simulink model "TosAeb"  (R2021a)
settings: Solver=FixedStepDiscrete  StartTime=0.0  StopTime=inf  FixedStep=0.01  RelTol=1e-3
undefined symbols (not defined in the file or in its folder): ACCModSts, AccSts1, AEB_ObjectMovingOrientation, AEB_ObjectMovingState, CN_BTCActive, CN_CurrentGearR, CN_CurrentGearR_DHT, CN_PTCActive, CN_VDCActive, CN_VSC_EspOff, CN_VSC_FCW_AEB_Error, CN_VSC_FCW_AEB_NoDispay, CN_VSC_FCW_AEB_PerfmDgdtn, CN_VSC_FCW_AEB_TempUnAvlbl, CN_VSC_VehStandstill, CNaccelAvoidMax, CNaccelAvoidMin, CNbrakePedalPress, CNcomfortStrLeftAccelMax, CNcomfortStrLeftAccelMin, CNcomfortStrRightAccelMax, CNcomfortStrRightAccelMin, CNDrvDoorOpen, CNDrvModeEco, CNDrvModeNorm, CNDrvModeOther, CNDrvModeSport, CNDrvSBRreminder, CNNull, CNobjInvalidID, CNPassDoorOpen, CNPassSBRreminder, CNPEBSAccelNegInf, CNPEBSAccelNull, CNPEBSTimeInf, CNPEBSTimeNull, CNSTOPMAX, CNSTOPNULL, CNsubjWidth, CNSwtOFF, CNSwtON, CNTTCMAX, CNTTCNULL, CNVehConfigAEBCycNone, CNVehConfigAEBNone, CNVehConfigAEBPedNone, CNVehConfigFCWNone, CNVehConfigJANone, CollisionSeverity, FALSE, FCWSnvty, JACycObjCalcInfoDefaultParams, JAPedObjCalcInfoDefaultParams, JAVehObjCalcInfoDefaultParams, latCycObjCalcInfoDefaultParams, latPedObjCalcInfoDefaultParams, longObjCalcInfoDefaultParams, ModSts, movingStatus, ObjectType ... (+1112)
libraries: simulink x3031, AEBSFunctionLib x50, Ta_Library x33, AEBSStateMachineLib x23, Mylibrary x12, simulink_extras x7, ObjSelect_lib x3, dspstat3 x2
(part 5 of 102)

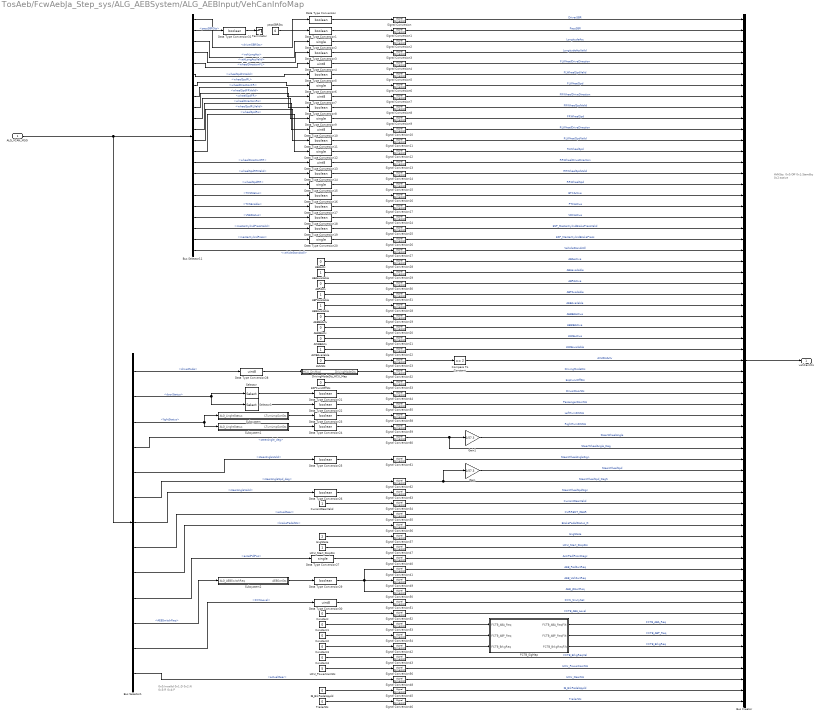
== system TosAeb/FcwAebJa_Step_sys/ALG_AEBSystem/ALG_AEBInput/VehCanInfoMap ==
  note: AVHSts:
      | 0x0:Off
      | 0x1:Standby
      | 0x2:active
  note: 0x0:Invailid
      | 0x1:D
      | 0x2:N
      | 0x3:R
      | 0x4:P
  ALG_VCAN_MSG : Inport  OutDataTypeStr=Bus: VehicleInfo_Struct  PortDimensions=1  BusOutputAsStruct=on
  ABAactv : Constant  Value=0  OutDataTypeStr=boolean
  ABAavailable : Constant  OutDataTypeStr=boolean
  ABPActv : Constant  Value=0  OutDataTypeStr=boolean
  ABPAvailable : Constant  OutDataTypeStr=boolean
  AEBAvailable : Constant  OutDataTypeStr=boolean
  AEBBAActv : Constant  Value=0  OutDataTypeStr=boolean
  AEBIBActv : Constant  Value=0  OutDataTypeStr=boolean
  AVHSts : Constant  Value=0  OutDataTypeStr=boolean
  AWBAvailable : Constant  OutDataTypeStr=boolean
  AWBBActv : Constant  Value=0  OutDataTypeStr=boolean
  Bus Creator : BusCreator  Inputs=63  OutDataTypeStr=Bus: BusVehCanInfo
      settings: InheritFromInputs=off
  Bus Selector1 : BusSelector  OutputSignals=bodyInfo.driveMode,bodyInfo.doorStatus,bodyInfo.lightStatus,chasssisInfo.steerAngle_deg,chasssisInfo.steerAngleValid,chasssisInfo.steerAngleSpd_deg,chasssisInfo.steerAngleValid,chasssisInfo.actualGear,chasssisInfo.brakePedalSts,chasssisInfo.accelPdlPos,bodyInfo.AEBSwitchReq,bodyInfo.FCWLevel,chasssisInfo.actualGear
  Bus Selector11 : BusSelector  OutputSignals=bodyInfo.driverSBRSts,bodyInfo.passSBRSts,chasssisInfo.vehLongAcc,chasssisInfo.vehLongAccValid,chasssisInfo.wheelDirectionFL,chasssisInfo.wheelSpdFLValid,chasssisInfo.wheelSpdFL,chasssisInfo.wheelDirectionFR,chasssisInfo.wheelSpdFRValid,chasssisInfo.wheelSpdFR,chasssisInfo.wheelDirectionRL,chasssisInfo.wheelSpdRLValid,chasssisInfo.wheelSpdRL,chasssisInfo.wheelDirectionRR,chasssisInfo.wheelSpdRRValid,chasssisInfo.wheelSpdRR,chasssisInfo.TCSStatus,chasssisInfo.TCSEnable,chasssisInfo.VSEStatus,chasssisInfo.masterCylindPressValid,chasssisInfo.masterCylindPress,chasssisInfo.vehicleStandstill
  Compare To Constant : simulink/Logic and Bit Operations/Compare To Constant  relop===  const=2  OutDataTypeStr=boolean
  Constant{,1..3} (4 alike) : Constant  Value=0  OutDataTypeStr=uint8
  Constant4 : Constant  Value=0  OutDataTypeStr=single
  CurrentGearValid : Constant  OutDataTypeStr=boolean
  Data Type Conversion{,1,3,5,8,11,14,16..19,21..26,29,31} (19 alike) : DataTypeConversion  OutDataTypeStr=boolean
  Data Type Conversion{4,7,10,13,28,30} (6 alike) : DataTypeConversion  OutDataTypeStr=uint8
  Data Type Conversion{2,6,9,12,15,20,27} (7 alike) : DataTypeConversion  OutDataTypeStr=single
  DrivingModeDis_HCU_Map : Subsystem  VariantControl=P_AEB_PowerTrainConfig == 1
  ESPFuncOffSts : Constant  Value=0  OutDataTypeStr=boolean
  EngState : Constant  Value=2  OutDataTypeStr=uint8
  FCTB_SigMap : Subsystem
  Gain : Gain  Gain=1/57.3
  Gain1 : Gain  Gain=1/57.3
  HCU_PowertrainSts : Constant  Value=5  OutDataTypeStr=uint8
  HCU_Start_StopSts : Constant  OutDataTypeStr=uint8
  IB_BrkPedalAppld : Constant  Value=0  OutDataTypeStr=boolean
  Selector : Selector  InputPortWidth=6  IndexOptions=Index vector (dialog)  Indices=1  OutputSizes=1
  Selector1 : Selector  InputPortWidth=6  IndexOptions=Index vector (dialog)  Indices=2  OutputSizes=1
  Signal Conversion{,1..37,40..56,58..63} (61 alike) : SignalConversion  OverrideOpt=off
  Subsystem : Subsystem
  Subsystem1 : Subsystem
  Subsystem2 : Subsystem
  Terminator : Terminator
  TrailerSts : Constant  Value=0  OutDataTypeStr=boolean
  passSBRSts : Constant  Value=0  OutDataTypeStr=boolean
  vehCanInfo : Outport  OutDataTypeStr=Bus: BusVehCanInfo
  wires:
  Bus Selector1/2 -> Selector1/1, Selector/1  "<doorStatus>"
  Subsystem2/1(AEBSwtSts) -> Data Type Conversion29/1
  Signal Conversion56/1 -> Bus Creator/60  "HCU_PowertrainSts"
  Data Type Conversion30/1 -> Signal Conversion51/1
  Bus Selector11/5 -> Data Type Conversion4/1  "<wheelDirectionFL>"
  Bus Selector1/12 -> Data Type Conversion30/1  "<FCWLevel>"
  Bus Selector1/11 -> Subsystem2/1(ALG_AEBSwitchReq)  "<AEBSwitchReq>"
  CurrentGearValid/1 -> Signal Conversion34/1
  Bus Selector1/9 -> Signal Conversion36/1  "<brakePedalSts>"
  Data Type Conversion21/1 -> Signal Conversion44/1
  Bus Selector1/1 -> Data Type Conversion28/1  "<driveMode>"
  Bus Selector11/10 -> Data Type Conversion9/1  "<wheelSpdFR>"
  Bus Selector11/19 -> Data Type Conversion18/1  "<VSEStatus>"
  Data Type Conversion27/1 -> Signal Conversion40/1
  Bus Selector1/13 -> Signal Conversion48/1  "<actualGear>"
  Bus Selector1/10 -> Data Type Conversion27/1  "<accelPdlPos>"
  Data Type Conversion24/1 -> Signal Conversion59/1
  Bus Selector11/13 -> Data Type Conversion12/1  "<wheelSpdRL>"
  Data Type Conversion26/1 -> Signal Conversion63/1
  Data Type Conversion15/1 -> Signal Conversion15/1
  Signal Conversion25/1 -> Bus Creator/20  "ESP_MasterCylindBrakePressValid"
  Data Type Conversion16/1 -> Signal Conversion16/1
  Data Type Conversion25/1 -> Signal Conversion61/1
  Data Type Conversion2/1 -> Signal Conversion2/1
  IB_BrkPedalAppld/1 -> Signal Conversion45/1
  Data Type Conversion12/1 -> Signal Conversion12/1
  Signal Conversion34/1 -> Bus Creator/45  "CurrentGearValid"
  Data Type Conversion23/1 -> Signal Conversion58/1
  Data Type Conversion22/1 -> Signal Conversion55/1
  HCU_PowertrainSts/1 -> Signal Conversion56/1
  Data Type Conversion20/1 -> Signal Conversion26/1
  HCU_Start_StopSts/1 -> Signal Conversion47/1
  EngState/1 -> Signal Conversion37/1
  Bus Selector1/6 -> Signal Conversion62/1  "<steerAngleSpd_deg>"
  Bus Selector1/4 -> Signal Conversion60/1  "<steerAngle_deg>"
  Bus Selector1/5 -> Data Type Conversion25/1  "<steerAngleValid>"
  Subsystem1/1(LTurnLmpSwtSts) -> Data Type Conversion24/1
  Subsystem/1(LTurnLmpSwtSts) -> Data Type Conversion23/1
  Data Type Conversion5/1 -> Signal Conversion5/1
  Signal Conversion16/1 -> Bus Creator/17  "BTCActive"
  Data Type Conversion18/1 -> Signal Conversion24/1
  Data Type Conversion19/1 -> Signal Conversion25/1
  Signal Conversion15/1 -> Bus Creator/16  "RRWheelSpd"
  Bus Selector11/7 -> Data Type Conversion6/1  "<wheelSpdFL>"
  Signal Conversion32/1 -> Bus Creator/33  "DrivingModeDis"
  Bus Selector11/15 -> Data Type Conversion14/1  "<wheelSpdRRValid>"
  Data Type Conversion4/1 -> Signal Conversion4/1
  Data Type Conversion17/1 -> Signal Conversion17/1
  Data Type Conversion7/1 -> Signal Conversion7/1
  Signal Conversion5/1 -> Bus Creator/6  "FLWheelSpdValid"
  Data Type Conversion14/1 -> Signal Conversion14/1
  Signal Conversion23/1 -> Compare To Constant/1
  Data Type Conversion28/1 -> DrivingModeDis_HCU_Map/1(HCU_DrvMod)
  Data Type Conversion10/1 -> Signal Conversion10/1
  Data Type Conversion13/1 -> Signal Conversion13/1
  Data Type Conversion11/1 -> Signal Conversion11/1
  Data Type Conversion8/1 -> Signal Conversion8/1
  Data Type Conversion9/1 -> Signal Conversion9/1
  Bus Selector11/21 -> Data Type Conversion20/1  "<masterCylindPress>"
  Data Type Conversion3/1 -> Signal Conversion3/1
  Data Type Conversion6/1 -> Signal Conversion6/1
  Data Type Conversion1/1 -> Signal Conversion1/1
  TrailerSts/1 -> Signal Conversion46/1
  Data Type Conversion/1 -> Signal Conversion/1
  Bus Selector11/11 -> Data Type Conversion10/1  "<wheelDirectionRL>"
  Bus Selector11/18 -> Data Type Conversion17/1  "<TCSEnable>"
  Selector1/1 -> Data Type Conversion22/1
  Bus Selector11/20 -> Data Type Conversion19/1  "<masterCylindPressValid>"
  Bus Selector11/22 -> Signal Conversion27/1  "<vehicleStandstill>"
  Bus Selector11/14 -> Data Type Conversion13/1  "<wheelDirectionRR>"
  Bus Selector11/16 -> Data Type Conversion15/1  "<wheelSpdRR>"
  Bus Selector11/17 -> Data Type Conversion16/1  "<TCSStatus>"
  Bus Selector11/12 -> Data Type Conversion11/1  "<wheelSpdRLValid>"
  Bus Selector11/8 -> Data Type Conversion7/1  "<wheelDirectionFR>"
  Signal Conversion48/1 -> Bus Creator/61  "HCU_GearSts"
  Bus Selector11/9 -> Data Type Conversion8/1  "<wheelSpdFRValid>"
  Bus Selector11/6 -> Data Type Conversion5/1  "<wheelSpdFLValid>"
  Bus Selector11/4 -> Data Type Conversion3/1  "<vehLongAccValid>"
  Bus Selector11/3 -> Data Type Conversion2/1  "<vehLongAcc>"
  Bus Selector11/1 -> Data Type Conversion/1  "<driverSBRSts>"
  ESPFuncOffSts/1 -> Signal Conversion33/1
  AVHSts/1 -> Signal Conversion23/1
  AWBBActv/1 -> Signal Conversion21/1
  AEBIBActv/1 -> Signal Conversion20/1
  AEBBAActv/1 -> Signal Conversion19/1
  AWBAvailable/1 -> Signal Conversion22/1
  AEBAvailable/1 -> Signal Conversion18/1
  ABPAvailable/1 -> Signal Conversion31/1
  ABPActv/1 -> Signal Conversion30/1
  ABAavailable/1 -> Signal Conversion29/1
  ABAactv/1 -> Signal Conversion28/1
  Constant4/1 -> Signal Conversion43/1
  Signal Conversion13/1 -> Bus Creator/14  "RRWheelDriveDirection"
  Constant3/1 -> Signal Conversion42/1
  Signal Conversion47/1 -> Bus Creator/49  "HCU_Start_StopSts"
  Signal Conversion46/1 -> Bus Creator/63  "TrailerSts"
  Signal Conversion43/1 -> Bus Creator/59  "FCTB_BrkgReqVal"
  Signal Conversion42/1 -> FCTB_SigMap/3(FCTB_BrkgReq)
  FCTB_SigMap/3(FCTB_BrkgReqFilt) -> Bus Creator/58  "FCTB_BrkgReq"
  FCTB_SigMap/1(FCTB_ABA_ReqFilt) -> Bus Creator/56  "FCTB_ABA_Req"
  Data Type Conversion29/1 -> Signal Conversion41/1, Signal Conversion50/1, Signal Conversion49/1
  FCTB_SigMap/2(FCTB_ABP_ReqFilt) -> Bus Creator/57  "FCTB_ABP_Req"
  Bus Selector1/3 -> Subsystem1/1(ALG_LinghtStatus), Subsystem/1(ALG_LinghtStatus)  "<lightStatus>"
  DrivingModeDis_HCU_Map/1(DrivingModeDis) -> Signal Conversion32/1
  Gain1/1 -> Bus Creator/39  "SteerWheelAngle"
  Gain/1 -> Bus Creator/42  "SteerWheelSpd"
  Compare To Constant/1 -> Bus Creator/32  "AVHStsActv"
  Signal Conversion61/1 -> Bus Creator/41  "SteerWheelAngleSign"
  Signal Conversion63/1 -> Bus Creator/44  "SteerWheelSpdSign"
  Signal Conversion58/1 -> Bus Creator/37  "LeftTurnSWSts"
  Signal Conversion59/1 -> Bus Creator/38  "RightTurnSWSts"
  Signal Conversion54/1 -> FCTB_SigMap/2(FCTB_ABP_Req)
  Signal Conversion55/1 -> Bus Creator/36  "PassengerDoorSts"
  Signal Conversion52/1 -> Bus Creator/55  "FCTB_ABA_Level"
  Signal Conversion53/1 -> FCTB_SigMap/1(FCTB_ABA_Req)
  Signal Conversion49/1 -> Bus Creator/52  "AEB_VehSwtReq"
  Signal Conversion30/1 -> Bus Creator/25  "ABPActive"
  Signal Conversion50/1 -> Bus Creator/53  "AEB_JASwtReq"
  Signal Conversion51/1 -> Bus Creator/54  "FCW_SnvtySet"
  Signal Conversion44/1 -> Bus Creator/35  "DriverDoorSts"
  Signal Conversion45/1 -> Bus Creator/62  "IB_BrkPedalAppld"
  Signal Conversion40/1 -> Bus Creator/50  "AccrPedlPosnDiagc"
  Signal Conversion60/1 -> Bus Creator/40, Gain1/1  "SteerWheelAngle_Deg"
  Signal Conversion41/1 -> Bus Creator/51  "AEB_PedSwtReq"
  Signal Conversion36/1 -> Bus Creator/47  "BrakePedalStatus_G"
  Signal Conversion37/1 -> Bus Creator/48  "EngState"
  Signal Conversion33/1 -> Bus Creator/34  "EspFuncOffSts"
  Signal Conversion35/1 -> Bus Creator/46  "CURRENT_GEAR"
  Signal Conversion31/1 -> Bus Creator/26  "ABPAvailable"
  Signal Conversion29/1 -> Bus Creator/24  "ABAavailable"
  Signal Conversion27/1 -> Bus Creator/22  "VehicleStandstill"
  Signal Conversion28/1 -> Bus Creator/23  "ABAactive"
  Signal Conversion26/1 -> Bus Creator/21  "ESP_MasterCylindBrakePress"
  Signal Conversion22/1 -> Bus Creator/31  "AWBavailable"
  Signal Conversion24/1 -> Bus Creator/19  "VDCActive"
  Signal Conversion21/1 -> Bus Creator/30  "AWBactive"
  Signal Conversion20/1 -> Bus Creator/29  "AEBIBActive"
  Signal Conversion18/1 -> Bus Creator/27  "AEBAvailable"
  Signal Conversion19/1 -> Bus Creator/28  "AEBBAActive"
  Signal Conversion17/1 -> Bus Creator/18  "PTCActive"
  Signal Conversion9/1 -> Bus Creator/10  "FRWheelSpd"
  Signal Conversion8/1 -> Bus Creator/9  "FRWheelSpdValid"
  Signal Conversion14/1 -> Bus Creator/15  "RRWheelSpdValid"
  Signal Conversion12/1 -> Bus Creator/13  "RLWheelSpd"
  Signal Conversion10/1 -> Bus Creator/11  "RLWheelDriveDirection"
  Signal Conversion11/1 -> Bus Creator/12  "RLWheelSpdValid"
  Signal Conversion6/1 -> Bus Creator/7  "FLWheelSpd"
  Signal Conversion4/1 -> Bus Creator/5  "FLWheelDriveDirection"
  Signal Conversion7/1 -> Bus Creator/8  "FRWheelDriveDirection"
  Signal Conversion2/1 -> Bus Creator/3  "LongitudeAcc"
  Signal Conversion3/1 -> Bus Creator/4  "LongitudeAccValid"
  Signal Conversion1/1 -> Bus Creator/2  "PassSBR"
  Signal Conversion/1 -> Bus Creator/1  "DriverSBR"
  Bus Creator/1 -> vehCanInfo/1
  Constant2/1 -> Signal Conversion54/1
  Constant1/1 -> Signal Conversion53/1
  Constant/1 -> Signal Conversion52/1
  Bus Selector1/8 -> Signal Conversion35/1  "<actualGear>"
  Bus Selector1/7 -> Data Type Conversion26/1  "<steerAngleValid>"
  Selector/1 -> Data Type Conversion21/1
  ALG_VCAN_MSG/1 -> Bus Selector1/1, Bus Selector11/1
  Signal Conversion62/1 -> Bus Creator/43, Gain/1  "SteerWheelSpd_DegS"
  Bus Selector11/2 -> Data Type Conversion31/1  "<passSBRSts>"
  passSBRSts/1 -> Data Type Conversion1/1
  Data Type Conversion31/1 -> Terminator/1

== system TosAeb/FcwAebJa_Step_sys/ALG_AEBSystem/ALG_AEBInput/VehConfigInfoMap ==
  Bus Creator : BusCreator  Inputs=6  OutDataTypeStr=Bus: BusVehConfigInfo
      settings: InheritFromInputs=off
  Constant{,1..5} (6 alike) : Constant  OutDataTypeStr=boolean
  Data Type Conversion{,1..5} (6 alike) : DataTypeConversion  OutDataTypeStr=boolean
  vehConfigInfo : Outport
  wires:
  Data Type Conversion5/1 -> Bus Creator/6
  Constant5/1 -> Data Type Conversion5/1
  Constant/1 -> Data Type Conversion1/1
  Constant1/1 -> Data Type Conversion/1
  Constant2/1 -> Data Type Conversion3/1
  Constant3/1 -> Data Type Conversion2/1
  Constant4/1 -> Data Type Conversion4/1
  Bus Creator/1 -> vehConfigInfo/1
  Data Type Conversion4/1 -> Bus Creator/5
  Data Type Conversion2/1 -> Bus Creator/4
  Data Type Conversion3/1 -> Bus Creator/3
  Data Type Conversion/1 -> Bus Creator/2
  Data Type Conversion1/1 -> Bus Creator/1

== system TosAeb/FcwAebJa_Step_sys/ALG_AEBSystem/ALG_AEBInput/subjInfoMap ==
  vxvRefMsFilt : Inport
  axvRefMs2 : Inport  Port=2
  ayvRefMs2 : Inport  Port=3
  kapTraj : Inport  Port=4
  psiDtOpt : Inport  Port=5
  Bus Creator : BusCreator  Inputs=5  OutDataTypeStr=Bus: BusSubjInfo
      settings: InheritFromInputs=off
  Signal Conversion{,1..4} (5 alike) : SignalConversion  OverrideOpt=off
  subjInfo : Outport  OutDataTypeStr=Bus: BusSubjInfo
  wires:
  Signal Conversion/1 -> Bus Creator/1  "subjVel"
  Signal Conversion1/1 -> Bus Creator/2  "subjLongAccel"
  Signal Conversion2/1 -> Bus Creator/3  "subjLatAccel"
  Signal Conversion3/1 -> Bus Creator/4  "subjCurvTraj"
  Signal Conversion4/1 -> Bus Creator/5  "subjYawRate"
  Bus Creator/1 -> subjInfo/1
  vxvRefMsFilt/1 -> Signal Conversion/1  "subjVel"
  axvRefMs2/1 -> Signal Conversion1/1  "subjLongAccel"
  psiDtOpt/1 -> Signal Conversion4/1  "subjYawRate"
  ayvRefMs2/1 -> Signal Conversion2/1  "subjLatAccel"
  kapTraj/1 -> Signal Conversion3/1  "subjCurvTraj"

== system TosAeb/FcwAebJa_Step_sys/ALG_AEBSystem/ALG_AEBInput/vxvRefMsFilt ==
  vxvRefMs : Inport  OutDataTypeStr=single
  VehSpd : Inport  Port=2
  Add : Sum  Inputs=+++
  Constant : Constant  Value=P_AEB_vxvref_Config
  Gain1 : Gain  Gain=1/3
  Switch : Switch  Criteria=u2 ~= 0
  Unit Delay : UnitDelay  SampleTime=-1  HasFrameUpgradeWarning=on
  Unit Delay1 : UnitDelay  SampleTime=-1  HasFrameUpgradeWarning=on
  vxvRefMsFilt : Outport
  wires:
  VehSpd/1 -> Unit Delay/1, Add/1
  Unit Delay/1 -> Unit Delay1/1, Add/2
  Unit Delay1/1 -> Add/3
  Add/1 -> Gain1/1
  Constant/1 -> Switch/2
  Gain1/1 -> Switch/3  "AEB_vxvRefMs_Can"
  vxvRefMs/1 -> Switch/1
  Switch/1 -> vxvRefMsFilt/1  "AEB_vxvRefMsFilt"

== system TosAeb/FcwAebJa_Step_sys/ALG_AEBSystem/ALG_AEBOutput/AEBS_BrkInfoCalc ==
  brkInfoInputSignals : Inport
  AEBS_BrkInfoMap : Subsystem
  AEB_BrkReqFuncCalc : Subsystem
  AEB_VehHldReqCalc : Subsystem
  Bus Selector : BusSelector  OutputSignals=AEBpartFuncImpArb,AEBfullFuncImpArb,EBAFuncImpArb,cycAEBpartFuncImpArb,cycAEBfullFuncImpArb,pedAEBfullFuncImpArb,JA_AEBfullFuncImpArb,JA_pedAEBRawModSts,FCTBFuncImpArb
  Bus Selector1 : BusSelector  OutputSignals=vehAEBpartRawModSts,vehAEBfullRawModSts,vehEBARawModSts,pedAEBfullRawModSts,cycAEBpartRawModSts,cycAEBfullRawModSts,JA_vehAEBRawModSts,JA_pedAEBRawModSts,AEBTrigger,JA_cycAEBRawModSts
  Bus Selector2 : BusSelector  OutputSignals=AEBTrigger,EBAFuncImpArb
  Bus Selector3 : BusSelector  OutputSignals=ABALvl,ABATrigger,AEBTrigger,axTgtReqValue,acuteWarningTrigger,acuteWarningLvl,ABPTrigger
  EBA_TgtDecel_ReqJudge : Subsystem
  AEB_BRK_INFO : Outport  OutDataTypeStr=Bus: AEB_BRK_INFO_BUS
  wires:
  AEBS_BrkInfoMap/1(AEB_BRK_INFO) -> AEB_BRK_INFO/1
  Bus Selector3/7 -> AEBS_BrkInfoMap/7(ABPTrigger)  "<ABPTrigger>"
  Bus Selector3/6 -> AEBS_BrkInfoMap/6(acuteWarningLvl)  "<acuteWarningLvl>"
  Bus Selector3/5 -> AEBS_BrkInfoMap/5(acuteWarningTrigger)  "<acuteWarningTrigger>"
  Bus Selector3/4 -> AEBS_BrkInfoMap/4(axTgtReqValue)  "<axTgtReqValue>"
  Bus Selector3/3 -> AEBS_BrkInfoMap/3(AEBTrigger)  "<AEBTrigger>"
  Bus Selector3/1 -> AEBS_BrkInfoMap/1(ABALvl)  "<ABALvl>"
  Bus Selector3/2 -> AEBS_BrkInfoMap/2(ABATrigger)  "<ABATrigger>"
  Bus Selector2/1 -> EBA_TgtDecel_ReqJudge/1(AEBtrigger)  "<AEBTrigger>"
  Bus Selector2/2 -> EBA_TgtDecel_ReqJudge/2(EBAFuncImpArb)  "<EBAFuncImpArb>"
  EBA_TgtDecel_ReqJudge/1(Inter_EBA_TgtDecel_Req) -> AEBS_BrkInfoMap/8(EBA_TgtDecel_Req)  "EBA_TgtDecel_Req"
  AEB_VehHldReqCalc/1(Inter_AEB_VehHldReq) -> AEBS_BrkInfoMap/9(AEB_VehHldReq)  "AEB_VehHldReq"
  AEB_BrkReqFuncCalc/1(AEB_BrkReqFunc) -> AEBS_BrkInfoMap/10(AEB_BrkReqFunc)  "AEB_BrkReqFunc"
  Bus Selector1/9 -> AEB_VehHldReqCalc/9(AEBtrigger)  "<AEBTrigger>"
  Bus Selector1/8 -> AEB_VehHldReqCalc/8(JA_pedAEBRawModSts)  "<JA_pedAEBRawModSts>"
  Bus Selector1/7 -> AEB_VehHldReqCalc/7(JA_vehAEBRawModSts)  "<JA_vehAEBRawModSts>"
  Bus Selector1/6 -> AEB_VehHldReqCalc/6(cycAEBfullRawModSts)  "<cycAEBfullRawModSts>"
  Bus Selector1/5 -> AEB_VehHldReqCalc/5(cycAEBpartRawModSts)  "<cycAEBpartRawModSts>"
  Bus Selector1/4 -> AEB_VehHldReqCalc/4(pedAEBfullRawModSts)  "<pedAEBfullRawModSts>"
  Bus Selector1/3 -> AEB_VehHldReqCalc/3(vehEBARawModSts)  "<vehEBARawModSts>"
  Bus Selector1/2 -> AEB_VehHldReqCalc/2(vehAEBfullRawModSts)  "<vehAEBfullRawModSts>"
  Bus Selector1/1 -> AEB_VehHldReqCalc/1(vehAEBpartRawModSts)  "<vehAEBpartRawModSts>"
  Bus Selector/9 -> AEB_BrkReqFuncCalc/9(FCTBFuncImpArb)  "<FCTBFuncImpArb>"
  Bus Selector/8 -> AEB_BrkReqFuncCalc/8(JA_pedAEBRawModSts)  "<JA_pedAEBRawModSts>"
  Bus Selector/7 -> AEB_BrkReqFuncCalc/7(JA_AEBfullFuncImpArb)  "<JA_AEBfullFuncImpArb>"
  Bus Selector/6 -> AEB_BrkReqFuncCalc/6(pedAEBfullFuncImpArb)  "<pedAEBfullFuncImpArb>"
  Bus Selector/5 -> AEB_BrkReqFuncCalc/5(cycAEBfullFuncImpArb)  "<cycAEBfullFuncImpArb>"
  Bus Selector/4 -> AEB_BrkReqFuncCalc/4(cycAEBpartFuncImpArb)  "<cycAEBpartFuncImpArb>"
  Bus Selector/3 -> AEB_BrkReqFuncCalc/3(EBAFuncImpArb)  "<EBAFuncImpArb>"
  Bus Selector/1 -> AEB_BrkReqFuncCalc/1(AEBpartFuncImpArb)  "<AEBpartFuncImpArb>"
  Bus Selector/2 -> AEB_BrkReqFuncCalc/2(AEBfullFuncImpArb)  "<AEBfullFuncImpArb>"
  brkInfoInputSignals/1 -> Bus Selector/1, Bus Selector1/1, Bus Selector2/1, Bus Selector3/1
  Bus Selector1/10 -> AEB_VehHldReqCalc/10(JA_cycAEBRawModSts)  "<JA_cycAEBRawModSts>"

== system TosAeb/FcwAebJa_Step_sys/ALG_AEBSystem/ALG_AEBOutput/AEBS_DVRInfoCalc ==
  VehicleConfigFCW : Inport
  VehicleConfigAEB : Inport  Port=2
  longObjCalcInfo : Inport  Port=3
  VehicleConfigAEBPed : Inport  Port=4
  latPedTgtCalcInfo : Inport  Port=5
  VehicleConfigAEBCyc : Inport  Port=6
  latCycTgtCalcInfo : Inport  Port=7
  JAVehTgtCalcInfo : Inport  Port=8
  VehConfigAEBJA : Inport  Port=9
  AEBJAPedTgtCalcInfo : Inport  Port=10
  AEB_DVR_INFOMap : Subsystem
  AEB_JAPED_DVR_INFO : Subsystem
  AEB_JAVEH_DVR_INFO : Subsystem
  AEB_LATCYC_DVR_INFO : Subsystem
  AEB_LATPED_DVR_INFO : Subsystem
  AEB_LON_DVR_INFO : Subsystem
  AEB_DVR_INFO : Outport  OutDataTypeStr=Bus: AEB_DVR_INFO_BUS
  wires:
  VehConfigAEBJA/1 -> AEB_JAVEH_DVR_INFO/2(VehConfigAEBJA), AEB_JAPED_DVR_INFO/1(VehConfigAEBJA)
  VehicleConfigAEBCyc/1 -> AEB_LATCYC_DVR_INFO/1(VehicleConfigAEBCyc)
  VehicleConfigAEBPed/1 -> AEB_LATPED_DVR_INFO/1(VehicleConfigAEBPed)
  AEBJAPedTgtCalcInfo/1 -> AEB_JAPED_DVR_INFO/2(JAPedTgtCalcInfo)
  JAVehTgtCalcInfo/1 -> AEB_JAVEH_DVR_INFO/1(JAVehTgtCalcInfo)
  latCycTgtCalcInfo/1 -> AEB_LATCYC_DVR_INFO/2(latCycTgtCalcInfo)
  latPedTgtCalcInfo/1 -> AEB_LATPED_DVR_INFO/2(latPedTgtCalcInfo)
  longObjCalcInfo/1 -> AEB_LON_DVR_INFO/3(longObjCalcInfo)
  AEB_DVR_INFOMap/1(AEB_DVR_INFO) -> AEB_DVR_INFO/1
  AEB_JAPED_DVR_INFO/1(AEB_JAPED_DVR_INFO) -> AEB_DVR_INFOMap/5(AEB_JAPED_DVR_INFO)
  AEB_JAVEH_DVR_INFO/1(AEB_JAVEH_DVR_INFO) -> AEB_DVR_INFOMap/4(AEB_JAVEH_DVR_INFO)
  AEB_LATCYC_DVR_INFO/1(AEB_LATCYC_DVR_INFO) -> AEB_DVR_INFOMap/3(AEB_LATCYC_DVR_INFO)
  AEB_LATPED_DVR_INFO/1(AEB_LATPED_DVR_INFO) -> AEB_DVR_INFOMap/2(AEB_LATPED_DVR_INFO)
  AEB_LON_DVR_INFO/1(AEB_LON_DVR_INFO) -> AEB_DVR_INFOMap/1(AEB_LON_DVR_INFO)
  VehicleConfigAEB/1 -> AEB_LON_DVR_INFO/2(VehicleConfigAEB)
  VehicleConfigFCW/1 -> AEB_LON_DVR_INFO/1(VehicleConfigFCW)
